simulink model "ofdm_txrx_alamouti"  (Simulink 6.3)
settings: Solver=ode45  StartTime=0.0  StopTime=45000  RelTol=1e-3
InitFcn:
  | ofdm_rx_alamouti_init
StartFcn:
  | ofdm_rx_alamouti_init
values from neighbouring files (symbols this model uses):
  CPLength = 16    [ofdm_tx_alamouti_init.m]
  CRCPolynomial = hex2dec('04c11db7')    [calcTxCRC.m]    (also in ofdm_tx_alamouti_init.m)
  hi = zeros(1,length(cci))    [ofdm_rx_alamouti_init.m]
  hr = zeros(1,length(ccr))    [ofdm_rx_alamouti_init.m]
  max_CP_length = 16    [ofdm_tx_alamouti_init.m]
  max_num_baseRateSymbols = 31    [ofdm_tx_alamouti_init.m]
  max_num_subcarriers = 64    [ofdm_tx_alamouti_init.m]
  max_num_trainingSymbols = 15    [ofdm_tx_alamouti_init.m]
  max_OFDM_symbols = 2047    [ofdm_tx_alamouti_init.m]
  modConstellation_qam16 = 0.75*[1 3 -1 -3]./3    [ofdm_tx_alamouti_init.m]
  modConstellation_qam256 = 0.9375*[3 1 5 7 11 9 13 15 -3 -1 -5 -7 -11 -9 -13 -15]./15    [ofdm_tx_alamouti_init.m]
  modConstellation_qam64 = 0.875*[3 1 5 7 -3 -1 -5 -7]./7    [ofdm_tx_alamouti_init.m]
  modConstellation_qpsk = [1 -1]./sqrt(2)    [ofdm_tx_alamouti_init.m]
  numBaseRateSymbols = 2    [ofdm_tx_alamouti_init.m]
  numFullRateSymbols = numFullRateSymbols + mod(numFullRateSymbols, 2)    [ofdm_tx_alamouti_init.m]
  numSubcarriers = 64    [ofdm_tx_alamouti_init.m]
  numTrainingSymbols = 4    [ofdm_tx_alamouti_init.m]
  pkt_crossCorr_thresh = 0.7    [ofdm_rx_alamouti_init.m]
  pkt_energy_thresh = 0    [ofdm_rx_alamouti_init.m]
  pkt_numPayloadBytes = 24+4+20+20+500    (= 568)    [ofdm_tx_alamouti_init.m]
  pktTiming_count_payloadStart = pktTiming_count_lsStart + pktTiming_ls_num*pktTiming_ls_length    [ofdm_rx_init_packetTimingControl.m]
  preamble = 6*[0 shortsyms_10 longsyms_2]    [ofdm_tx_alamouti_init.m]
  preamble_I = real(preamble)    [ofdm_tx_alamouti_init.m]
  preamble_Q = imag(preamble)    [ofdm_tx_alamouti_init.m]
  RAM_init_size = 4096    [ofdm_tx_alamouti_init.m]
  rx_longCorrCountLoad = 251-16    (= 235)    [ofdm_rx_alamouti_init.m]
  rx_longCorrThresh = 3000    [ofdm_rx_alamouti_init.m]
  RxDataScrambling_Seq = [40 198 78 63 82 173 102 245 48 111 172 115 147 230 216 93 72 65 62 2 205 242 122 90 128 83 105 97 73 10 5 252]    [ofdm_tx_alamouti_init.m]
  simOnly_numSamples = length(preamble)+( (numSubcarriers+CPLength)*(numTrainingSymbols + numBaseRateSymbols + numFullRateSymbols) )    [ofdm_tx_alamouti_init.m]
  train = [train train]    [ofdm_tx_alamouti_init.m]
  TxDataScrambling_Seq = [40 198 78 63 82 173 102 245 48 111 172 115 147 230 216 93 72 65 62 2 205 242 122 90 128 83 105 97 73 10 5 252]    [ofdm_tx_alamouti_init.m]
undefined symbols (not defined in the file or in its folder): CRC_table_gen, gcbh, interpFilter_4, xlBlockLoadCallback
libraries: xbsIndex_r4 x4373, dspmlti4 x4, dspsigops x4, commchan3 x2, simulink x2, commrflib2 x2, commsink2 x2, dspsrcs4 x1
(part 7 of 39)

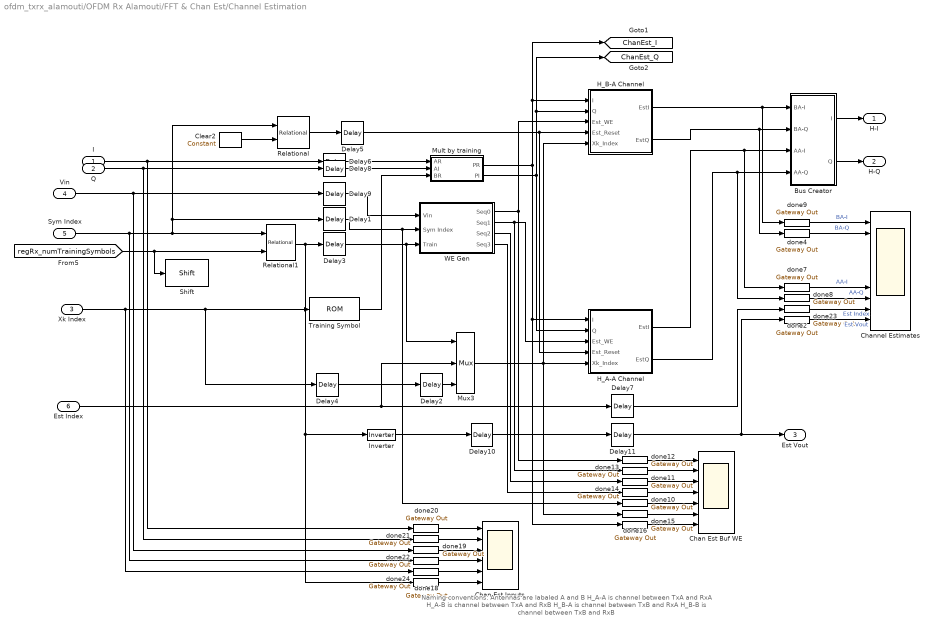
== system ofdm_txrx_alamouti/OFDM Rx Alamouti/FFT & Chan Est/Channel Estimation ==
  note: Naming conventions:
      | Antennas are labaled A and B
      | H_A-A is channel between TxA and RxA
      | H_A-B is channel between TxA and RxB
      | H_B-A is channel between TxB and RxA
      | H_B-B is channel between TxB and RxB
  I : Inport
  Q : Inport  Port=2
  Xk Index : Inport  Port=3
  Vin : Inport  Port=4
  Sym Index : Inport  Port=5
  Est Index : Inport  Port=6
  Bus Creator : Subsystem
  Chan Est Buf WE : Scope  NumInputPorts=7
      settings: TimeRange=2000  YMin=0~0~0~0~0~0~-0.4  YMax=1~1~1~1~30~70~0.4  SaveName=ScopeData21
  Chan Est Inputs : Scope  NumInputPorts=6
      settings: TimeRange=1800  YMin=-0.6~-0.4~0~5~0~-1  YMax=0.5~0.4~1~12~70~1  SaveName=ScopeData23
  Channel Estimates : Scope  NumInputPorts=10
      settings: TimeRange=3000  YMin=-1~-1~-1~-1~-1~-1~-1~-1~-1~0  YMax=1~1~1~1~1~1~1~1~1~1  SaveName=ScopeData24
  Clear2 : xbsIndex_r4/Constant  arith_type=Unsigned  const=1  n_bits=2  bin_pt=0  explicit_period=off  period=1  equ=P=C  opselect=C  inp2=PCIN>>17  opr=+  inp1=P  carry=CIN  dbl_ovrd=off  block_type=constant
      settings: block_version=VER_STRING_GOES_HERE
  Delay1 : xbsIndex_r4/Delay  en=off  latency=1  dbl_ovrd=off  reg_retiming=on  block_type=delay
      settings: infoedit=Hardware notes: A delay line is a chain, each link of which is an SRL16 followed by a flip-flop. If register retiming is enabled, the delay line is a chain of flip-flops.  xl_area=[0, 0, 0, 0, 0, 0, 0]  block_version=VER_STRING_GOES_HERE
  Delay{2,4..11} (9 alike) : xbsIndex_r4/Delay  en=off  latency=1  dbl_ovrd=off  reg_retiming=off  block_type=delay
      settings: infoedit=Hardware notes: A delay line is a chain, each link of which is an SRL16 followed by a flip-flop. If register retiming is enabled, the delay line is a chain of flip-flops.  xl_area=[0, 0, 0, 0, 0, 0, 0]  block_version=VER_STRING_GOES_HERE
  Delay3 : xbsIndex_r4/Delay  en=off  latency=1  dbl_ovrd=off  reg_retiming=on  block_type=delay
      settings: infoedit=Hardware notes: A delay line is a chain, each link of which is an SRL16 followed by a flip-flop. If register retiming is enabled, the delay line is a chain of flip-flops.  xl_area=[0, 0, 0, 0, 0, 0, 0]  block_version=VER_STRING_GOES_HERE
  H_A-A Channel : Subsystem
  H_B-A Channel : Subsystem
  Inverter : xbsIndex_r4/Inverter  en=off  latency=0  dbl_ovrd=off  block_type=inv
      settings: block_version=VER_STRING_GOES_HERE
  Mult by training : Subsystem
  Mux3 : xbsIndex_r4/Mux  inputs=2  en=off  latency=0  precision=Full  arith_type="Signed  (2's comp)"  n_bits=8  bin_pt=2  quantization=Truncate  overflow=Wrap  dbl_ovrd=off  block_type=mux
      settings: xl_area=[0, 0, 0, 0, 0, 0, 0]  block_version=VER_STRING_GOES_HERE
  Relational : xbsIndex_r4/Relational  mode=a<=b  en=off  latency=0  dbl_ovrd=off  block_type=relational
      settings: xl_area=[0, 0, 0, 0, 0, 0, 0]  block_version=VER_STRING_GOES_HERE
  Relational1 : xbsIndex_r4/Relational  mode=a<b  en=off  latency=0  dbl_ovrd=off  block_type=relational
      settings: xl_area=[3 0 0 6 0 0 0]  block_version=VER_STRING_GOES_HERE
  Shift : xbsIndex_r4/Shift  shift_dir=Left  shift_bits=1  en=off  latency=0  precision=User Defined  arith_type=Unsigned  n_bits=1+ceil(log2(max_num_trainingSymbols))  bin_pt=0  quantization=Truncate  overflow=Flag as error  dbl_ovrd=off  block_type=shift
      settings: block_version=VER_STRING_GOES_HERE
  Training Symbol : xbsIndex_r4/ROM  depth=length(train)/2  initVector=sign(train)  distributed_mem=Block RAM  rst=off  init_reg=0  en=off  latency=1  arith_type="Signed  (2's comp)"  n_bits=2  bin_pt=1  dbl_ovrd=off  optimize=Area  use_rpm=off  block_type=sprom
      settings: xl_area=[0, 0, 0, 0, 0, 0, 0]  block_version=VER_STRING_GOES_HERE
  WE Gen : Subsystem
  done{2,4,7..16,18..24} (19 alike) : xbsIndex_r4/Gateway Out  hdl_port=off  timing_constraint=None  locs_specified=off  LOCs={}  block_type=gatewayout
      settings: infoedit="Gateway out block.  Converts Xilinx fixed point inputs into ouputs of type Simulink integer, double, or fixed point.<P><P>Hardware notes:  In hardware these blocks become top level output ports or are discarded, depending on how they are configured."  xl_area=[0 0 0 0 1 0 0]  block_version=VER_STRING_GOES_HERE
  H-I : Outport
  H-Q : Outport  Port=2
  Est Vout : Outport  Port=3
  tag scope: ChanEst_I=global, ChanEst_Q=global
  wires:
  Training Symbol/1 -> Mult by training/3(BR)
  Delay4/1 -> Delay2/1
  I/1 -> Delay6/1, done20/1
  Q/1 -> Delay8/1, done21/1
  Sym Index/1 -> Relational1/1, Delay1/1, Relational/1, done22/1
  Xk Index/1 -> Delay4/1, Training Symbol/1, done18/1
  Clear2/1 -> Relational/2
  Relational/1 -> Delay5/1
  Mult by training/1(PR) -> done15/1, H_A-A Channel/1(I), H_B-A Channel/1(I), [ChanEst_I]
  Mux3/1 -> done16/1, H_A-A Channel/5(Xk_Index), H_B-A Channel/5(Xk_Index)
  Delay5/1 -> H_B-A Channel/4(Est_Reset), H_A-A Channel/4(Est_Reset)
  WE Gen/1(Seq0) -> H_B-A Channel/3(Est_WE), done12/1
  WE Gen/3(Seq2) -> done11/1
  WE Gen/4(Seq3) -> done14/1
  WE Gen/2(Seq1) -> done13/1, H_A-A Channel/3(Est_WE)
  Relational1/1 -> Delay3/1, done24/1, Inverter/1
  Mult by training/2(PI) -> H_B-A Channel/2(Q), [ChanEst_Q], H_A-A Channel/2(Q)
  done10/1 -> Chan Est Buf WE/5
  done12/1 -> Chan Est Buf WE/1
  done13/1 -> Chan Est Buf WE/2
  done11/1 -> Chan Est Buf WE/3
  done14/1 -> Chan Est Buf WE/4
  done16/1 -> Chan Est Buf WE/6
  done15/1 -> Chan Est Buf WE/7
  [regRx_numTrainingSymbols] -> Shift/1, Relational1/2
  Delay1/1 -> WE Gen/2(Sym Index), done10/1
  Delay3/1 -> WE Gen/3(Train), Mux3/1
  Delay6/1 -> Mult by training/1(AR)
  Delay8/1 -> Mult by training/2(AI)
  Vin/1 -> Delay9/1, done19/1
  Delay9/1 -> WE Gen/1(Vin)
  done18/1 -> Chan Est Inputs/5
  done20/1 -> Chan Est Inputs/1
  done21/1 -> Chan Est Inputs/2
  done19/1 -> Chan Est Inputs/3
  done22/1 -> Chan Est Inputs/4
  done24/1 -> Chan Est Inputs/6
  Delay7/1 -> done2/1
  Inverter/1 -> Delay10/1
  Delay11/1 -> done23/1, Est Vout/1
  H_B-A Channel/1(EstI) -> done9/1, Bus Creator/1(BA-I)
  H_B-A Channel/2(EstQ) -> done4/1, Bus Creator/2(BA-Q)
  H_A-A Channel/2(EstQ) -> done8/1, Bus Creator/4(AA-Q)
  ? -> Channel Estimates/6
  done4/1 -> Channel Estimates/2  "BA-Q"
  ? -> Channel Estimates/3
  ? -> Channel Estimates/4
  ? -> Channel Estimates/5
  done7/1 -> Channel Estimates/7  "AA-I"
  done8/1 -> Channel Estimates/8  "AA-Q"
  done2/1 -> Channel Estimates/9  "Est Index"
  done9/1 -> Channel Estimates/1  "BA-I"
  done23/1 -> Channel Estimates/10  "Est Vout"
  Bus Creator/1(I) -> H-I/1
  Bus Creator/2(Q) -> H-Q/1
  Delay10/1 -> Delay11/1
  Est Index/1 -> Delay7/1, Mux3/2
  Delay2/1 -> Mux3/3
  H_A-A Channel/1(EstI) -> done7/1, Bus Creator/3(AA-I)

== system ofdm_txrx_alamouti/OFDM Rx Alamouti/FFT & Chan Est/FFT ==
  xn_I : Inport
  xn_Q : Inport  Port=2
  Start : Inport  Port=3
  Constant3 : xbsIndex_r4/Constant  arith_type=Boolean  const=1  n_bits=1  bin_pt=0  explicit_period=off  period=1  equ=P=C  opselect=C  inp2=PCIN>>17  opr=+  inp1=P  carry=CIN  dbl_ovrd=off  block_type=constant
      settings: block_version=VER_STRING_GOES_HERE
  Constant4 : xbsIndex_r4/Constant  arith_type=Boolean  const=1  n_bits=1  bin_pt=0  explicit_period=off  period=1  equ=P=C  opselect=C  inp2=PCIN>>17  opr=+  inp1=P  carry=CIN  dbl_ovrd=off  block_type=constant
      settings: block_version=VER_STRING_GOES_HERE
  Constant6 : xbsIndex_r4/Constant  arith_type=Boolean  const=1  n_bits=1  bin_pt=0  explicit_period=off  period=1  equ=P=C  opselect=C  inp2=PCIN>>17  opr=+  inp1=P  carry=CIN  dbl_ovrd=off  block_type=constant
      settings: block_version=VER_STRING_GOES_HERE
  Convert : xbsIndex_r4/Convert  arith_type="Signed  (2's comp)"  n_bits=16  bin_pt=15  quantization=Truncate  overflow=Flag as error  latency=0  dbl_ovrd=off  pipeline=off  block_type=convert
      settings: block_version=VER_STRING_GOES_HERE
  Convert1 : xbsIndex_r4/Convert  arith_type="Signed  (2's comp)"  n_bits=16  bin_pt=15  quantization=Truncate  overflow=Flag as error  latency=0  dbl_ovrd=off  pipeline=off  block_type=convert
      settings: block_version=VER_STRING_GOES_HERE
  FFT Input : Scope  NumInputPorts=4
      settings: TimeRange=4000  YMin=-0.1~-0.2~0~0  YMax=0.15~0.8~1~80  SaveName=ScopeData14
  FFT Output : Scope  NumInputPorts=6
      settings: TimeRange=12000  YMin=-0.75~-0.75~0~0~0~-1  YMax=0.75~0.75~70~1~1~1  SaveName=ScopeData15
  FFTx : xbsIndex_r4/FFT v3_1   arch=Pipelined Streaming I/O  npts=64  scaling=Scaled  rounding=Convergent Rounding  tf_width=16  en_n=off  has_ovflo=on  rst=on  en=off  op_order=Natural Order  dbl_ovrd=off  explicit_period=off  period=1  use_dsp48=off  twiddle_mem_type=Block RAM  data_mem_type=Block RAM  num_bram_stages=3  block_type=fftx
      settings: infoedit=Devices Supported: Virtex-4, Virtex-II, Virtex-IIPro, Spartan-3 and Spartan-3E  xl_area=[0, 0, 0, 0, 0, 0, 0]  block_version=VER_STRING_GOES_HERE
  done{1..10} (10 alike) : xbsIndex_r4/Gateway Out  hdl_port=off  timing_constraint=None  locs_specified=off  LOCs={}  block_type=gatewayout
      settings: infoedit="Gateway out block.  Converts Xilinx fixed point inputs into ouputs of type Simulink integer, double, or fixed point.<P><P>Hardware notes:  In hardware these blocks become top level output ports or are discarded, depending on how they are configured."  xl_area=[0 0 0 0 1 0 0]  block_version=VER_STRING_GOES_HERE
  xk_I : Outport
  xk_Q : Outport  Port=2
  xk_Index : Outport  Port=3
  Vout : Outport  Port=4
  Done : Outport  Port=5
  xn_Index : Outport  Port=6
  wires:
  Constant3/1 -> FFTx/4
  Constant4/1 -> FFTx/5
  [rx_global_reset] -> FFTx/8
  xn_I/1 -> Convert/1
  FFTx/1 -> xk_I/1, done8/1
  FFTx/2 -> xk_Q/1, done9/1
  xn_Q/1 -> Convert1/1
  FFTx/3 -> xn_Index/1, done6/1
  Start/1 -> FFTx/3, done3/1
  FFTx/4 -> xk_Index/1, done7/1
  FFTx/7 -> Vout/1, done10/1
  Convert/1 -> FFTx/1, done4/1
  Convert1/1 -> FFTx/2, done5/1
  done4/1 -> FFT Input/1
  done5/1 -> FFT Input/2
  done3/1 -> FFT Input/3
  done6/1 -> FFT Input/4
  done8/1 -> FFT Output/1
  done9/1 -> FFT Output/2
  done7/1 -> FFT Output/3
  done10/1 -> FFT Output/4
  done2/1 -> FFT Output/5
  FFTx/9 -> done2/1, Done/1
  done1/1 -> FFT Output/6
  Constant6/1 -> FFTx/7
  [Rx_FFTScaling] -> FFTx/6
  FFTx/10 -> done1/1

== system ofdm_txrx_alamouti/OFDM Rx Alamouti/FFT & Chan Est/Input Buffers ==
  AntA I : Inport
  AntA Q : Inport  Port=2
  We : Inport  Port=3
  Wr Addr : Inport  Port=4
  Rd Addr : Inport  Port=5
  Ant Sel : Inport  Port=6
  Wr Slot : Inport  Port=7
  Antenna A RAM : Subsystem
  Antenna B RAM : Subsystem
  Inverter1 : xbsIndex_r4/Inverter  en=off  latency=0  dbl_ovrd=off  block_type=inv
      settings: block_version=VER_STRING_GOES_HERE
  Logical : xbsIndex_r4/Logical  logical_function=AND  inputs=2  en=off  latency=0  precision=Full  arith_type=Unsigned  n_bits=16  bin_pt=0  align_bp=on  dbl_ovrd=off  block_type=logical
      settings: block_version=8.2.02
  Logical1 : xbsIndex_r4/Logical  logical_function=AND  inputs=2  en=off  latency=0  precision=Full  arith_type=Unsigned  n_bits=16  bin_pt=0  align_bp=on  dbl_ovrd=off  block_type=logical
      settings: block_version=8.2.02
  Mux : xbsIndex_r4/Mux  inputs=2  en=off  latency=1  precision=Full  arith_type=Unsigned  n_bits=16  bin_pt=14  quantization=Truncate  overflow=Wrap  dbl_ovrd=off  block_type=mux
      settings: block_version=VER_STRING_GOES_HERE
  Mux1 : xbsIndex_r4/Mux  inputs=2  en=off  latency=1  precision=Full  arith_type=Unsigned  n_bits=16  bin_pt=14  quantization=Truncate  overflow=Wrap  dbl_ovrd=off  block_type=mux
      settings: block_version=VER_STRING_GOES_HERE
  Register : xbsIndex_r4/Register  init=0  rst=off  en=off  dbl_ovrd=off  block_type=register
      settings: block_version=VER_STRING_GOES_HERE
  Register1 : xbsIndex_r4/Register  init=0  rst=off  en=off  dbl_ovrd=off  block_type=register
      settings: block_version=VER_STRING_GOES_HERE
  Rx Buffers : Scope  NumInputPorts=6
      settings: TimeRange=2500  YMin=-0.015~-0.015~0~0~0~-5  YMax=0.015~0.015~80~80~1~5  SaveName=ScopeData19
  Rx Buffers Out : Scope  NumInputPorts=7
      settings: TimeRange=2500  YMin=-0.2~-0.7~0~-0.2~-0.7~-0.2~0  YMax=0.5~0.3~0.5~0.5~0~0.25~1  SaveName=ScopeData32
  done{1..13} (13 alike) : xbsIndex_r4/Gateway Out  hdl_port=off  timing_constraint=None  locs_specified=off  LOCs={}  block_type=gatewayout
      settings: infoedit="Gateway out block.  Converts Xilinx fixed point inputs into ouputs of type Simulink integer, double, or fixed point.<P><P>Hardware notes:  In hardware these blocks become top level output ports or are discarded, depending on how they are configured."  xl_area=[0 0 0 0 1 0 0]  block_version=VER_STRING_GOES_HERE
  " I" : Outport
  " Q" : Outport  Port=2
  wires:
  AntA I/1 -> Antenna A RAM/1(I), done8/1, Antenna B RAM/1(I)
  AntA Q/1 -> Antenna A RAM/2(Q), done9/1, Antenna B RAM/2(Q)
  Wr Addr/1 -> Antenna A RAM/4(Wr Addr), done7/1, Antenna B RAM/4(Wr Addr)
  Rd Addr/1 -> Antenna A RAM/5(Rd Addr), done10/1, Antenna B RAM/5(Rd Addr)
  Antenna A RAM/1(" I") -> Mux/2, done12/1
  Antenna A RAM/2(" Q") -> Mux1/2, done6/1
  Antenna B RAM/2(" Q") -> Mux1/3, done5/1
  Antenna B RAM/1(" I") -> Mux/3, done4/1
  Ant Sel/1 -> Mux/1, Mux1/1, done11/1
  Mux/1 -> Register/1
  Mux1/1 -> Register1/1
  Register/1 -> " I"/1, done3/1
  Register1/1 -> " Q"/1, done13/1
  done8/1 -> Rx Buffers/1  "AntA I"
  done9/1 -> Rx Buffers/2  "AntQ Q"
  done7/1 -> Rx Buffers/3  "Write Addr"
  done10/1 -> Rx Buffers/4  "Rd Addr"
  done2/1 -> Rx Buffers/5  "Write En A"
  done1/1 -> Rx Buffers/6  "Write En B"
  done11/1 -> Rx Buffers Out/1
  done12/1 -> Rx Buffers Out/2
  done6/1 -> Rx Buffers Out/3
  done4/1 -> Rx Buffers Out/4
  done5/1 -> Rx Buffers Out/5
  done3/1 -> Rx Buffers Out/6
  done13/1 -> Rx Buffers Out/7
  We/1 -> Logical/1, Logical1/1
  Wr Slot/1 -> Logical/2, Inverter1/1
  Inverter1/1 -> Logical1/2
  Logical/1 -> Antenna A RAM/3(We), done2/1
  Logical1/1 -> Antenna B RAM/3(We), done1/1

== system ofdm_txrx_alamouti/OFDM Rx Alamouti/FFT & Chan Est/Output Buffers ==
  I : Inport
  Q : Inport  Port=2
  Wr Addr : Inport  Port=3
  FFT Vout : Inport  Port=4
  SymIndex : Inport  Port=5
  Rd Addr : Inport  Port=6
  AntA Buffer : Subsystem
  AntB Buffer : Subsystem
  Bus Creator1 : BusCreator  Inputs=2
  Bus Creator2 : BusCreator  Inputs=2
  FFT Output Buffers : Scope  NumInputPorts=10
      settings: TimeRange=5000  YMin=-0.5~-0.5~0~0~0~0~-0.5~-1~0~0  YMax=0.5~0.5~100~1~1~100~0.5~1~0.02~1  SaveName=ScopeData53
  Inverter : xbsIndex_r4/Inverter  en=off  latency=0  dbl_ovrd=off  block_type=inv
      settings: block_version=VER_STRING_GOES_HERE
  LSB : xbsIndex_r4/Slice  nbits=1  boolean_output=on  mode=Upper Bit Location + Width  bit1=0  base1=LSB of Input  bit0=0  base0=LSB of Input  dbl_ovrd=off  block_type=slice
      settings: infoedit="Extracts a given range of bits from each input sample and presents it at the output.  The output type is ordinarily unsigned with binary point at zero, but can be Boolean when the slice is one bit wide.<P><P>Hardware notes: In hardware this block costs nothing."  block_version=VER_STRING_GOES_HERE
  Logical1 : xbsIndex_r4/Logical  logical_function=AND  inputs=2  en=off  latency=0  precision=Full  arith_type=Unsigned  n_bits=8  bin_pt=2  align_bp=on  dbl_ovrd=off  block_type=logical
      settings: xl_area=[0, 0, 0, 0, 0, 0, 0]  block_version=VER_STRING_GOES_HERE
  Logical2 : xbsIndex_r4/Logical  logical_function=AND  inputs=2  en=off  latency=0  precision=Full  arith_type=Unsigned  n_bits=8  bin_pt=2  align_bp=on  dbl_ovrd=off  block_type=logical
      settings: xl_area=[0, 0, 0, 0, 0, 0, 0]  block_version=VER_STRING_GOES_HERE
  done{1..10} (10 alike) : xbsIndex_r4/Gateway Out  hdl_port=off  timing_constraint=None  locs_specified=off  LOCs={}  block_type=gatewayout
      settings: infoedit="Gateway out block.  Converts Xilinx fixed point inputs into ouputs of type Simulink integer, double, or fixed point.<P><P>Hardware notes:  In hardware these blocks become top level output ports or are discarded, depending on how they are configured."  xl_area=[0 0 0 0 1 0 0]  block_version=VER_STRING_GOES_HERE
  " I" : Outport
  " Q" : Outport  Port=2
  wires:
  Rd Addr/1 -> AntA Buffer/5(Rd Addr), AntB Buffer/5(Rd Addr), done1/1
  SymIndex/1 -> LSB/1
  Logical2/1 -> AntA Buffer/3(We), done2/1
  Logical1/1 -> AntB Buffer/3(We), done10/1
  LSB/1 -> Inverter/1, Logical2/1
  AntB Buffer/1(" I") -> done3/1, Bus Creator1/2
  AntB Buffer/2(" Q") -> done4/1, Bus Creator2/2
  AntA Buffer/1(" I") -> done5/1, Bus Creator1/1
  AntA Buffer/2(" Q") -> done6/1, Bus Creator2/1
  FFT Vout/1 -> Logical2/2, Logical1/2
  Wr Addr/1 -> AntA Buffer/4(Wr Addr), AntB Buffer/4(Wr Addr), done7/1
  I/1 -> AntB Buffer/1(I), AntA Buffer/1(I), done8/1
  Q/1 -> AntB Buffer/2(Q), AntA Buffer/2(Q), done9/1
  done8/1 -> FFT Output Buffers/1  "I"
  done9/1 -> FFT Output Buffers/2  "Q"
  done7/1 -> FFT Output Buffers/3  "Wr Addr"
  done10/1 -> FFT Output Buffers/5  "B WE"
  done2/1 -> FFT Output Buffers/4  "A WE"
  done1/1 -> FFT Output Buffers/6  "Rd Addr"
  done3/1 -> FFT Output Buffers/9  "B_I"
  done4/1 -> FFT Output Buffers/10  "B_Q"
  done5/1 -> FFT Output Buffers/7  "A_I"
  done6/1 -> FFT Output Buffers/8  "A_Q"
  Bus Creator1/1 -> " I"/1
  Bus Creator2/1 -> " Q"/1
  Inverter/1 -> Logical1/1

== system ofdm_txrx_alamouti/OFDM Rx Alamouti/FFT & Chan Est/Symbol Counter ==
  note: SR Latch enables counter after the first symbol. This keeps
      | the symbol index at zero after the first FFT finishes so that
      | the symbol index, like xn and xk_index, is zero-indexed.
  FFT Done : Inport
  Payload : Inport  Port=2
  Counter : xbsIndex_r4/Counter  cnt_type=Free Running  cnt_to=Inf  operation=Up  start_count=0  cnt_by_val=1  arith_type=Unsigned  n_bits=ceil(log2(max_OFDM_symbols+1))  bin_pt=0  load_pin=off  rst=on  en=on  explicit_period=off  period=1  dbl_ovrd=off  use_behavioral_HDL=off  use_rpm=on  block_type=counter
      settings: xl_area=[6 12 0 12 0 0 0]  block_version=VER_STRING_GOES_HERE
  Logical1 : xbsIndex_r4/Logical  logical_function=AND  inputs=2  en=off  latency=0  precision=Full  arith_type=Unsigned  n_bits=8  bin_pt=2  align_bp=on  dbl_ovrd=off  block_type=logical
      settings: xl_area=[0, 0, 0, 0, 0, 0, 0]  block_version=VER_STRING_GOES_HERE
  Logical2 : xbsIndex_r4/Logical  logical_function=OR  inputs=2  en=off  latency=0  precision=Full  arith_type=Unsigned  n_bits=8  bin_pt=2  align_bp=on  dbl_ovrd=off  block_type=logical
      settings: xl_area=[4 0 0 4 0 0 0]  block_version=VER_STRING_GOES_HERE
  Posedge Det : Subsystem
  S-R Latch : Subsystem
  Sym Index : Outport
  wires:
  FFT Done/1 -> Logical1/1, S-R Latch/1(S)
  Counter/1 -> Sym Index/1
  Logical1/1 -> Counter/2
  S-R Latch/1(D) -> Logical1/2
  Logical2/1 -> Counter/1, S-R Latch/2(R)
  Posedge Det/1(Q) -> Logical2/1
  [rx_global_reset] -> Logical2/2
  Payload/1 -> Posedge Det/1(D)

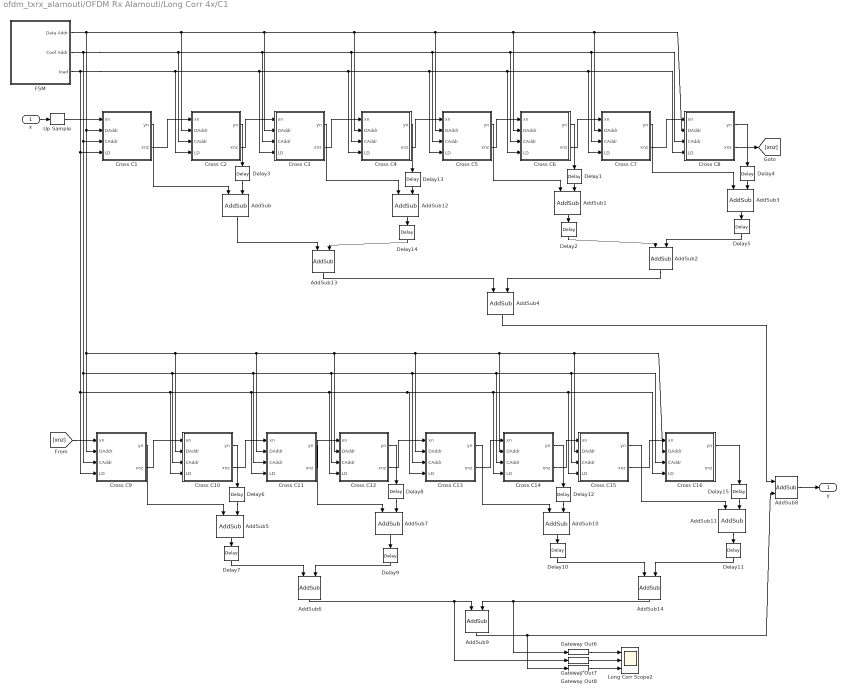
== system ofdm_txrx_alamouti/OFDM Rx Alamouti/Long Corr 4x/C1 ==
  x : Inport
  AddSub : xbsIndex_r4/AddSub  Orientation=down  mode=Addition  use_carryin=off  use_carryout=off  en=off  latency=0  precision=Full  arith_type=Unsigned  n_bits=8  bin_pt=2  quantization=Truncate  overflow=Wrap  dbl_ovrd=off  use_behavioral_HDL=on  pipelined=off  use_rpm=off  block_type=addsub
      settings: xl_area=[3 0 0 4 0 0 0]  block_version=VER_STRING_GOES_HERE
  AddSub{1..7,9..14} (13 alike) : xbsIndex_r4/AddSub  Orientation=down  mode=Addition  use_carryin=off  use_carryout=off  en=off  latency=1  precision=Full  arith_type=Unsigned  n_bits=8  bin_pt=2  quantization=Truncate  overflow=Wrap  dbl_ovrd=off  use_behavioral_HDL=on  pipelined=off  use_rpm=off  block_type=addsub
      AddSub1 settings: xl_area=[3 5 0 4 0 0 0]  block_version=VER_STRING_GOES_HERE
      AddSub10 settings: xl_area=[3 5 0 4 0 0 0]  block_version=VER_STRING_GOES_HERE
      AddSub11 settings: xl_area=[3 5 0 4 0 0 0]  block_version=VER_STRING_GOES_HERE
      AddSub12 settings: xl_area=[3 5 0 4 0 0 0]  block_version=VER_STRING_GOES_HERE
      AddSub13 settings: xl_area=[3 6 0 5 0 0 0]  block_version=VER_STRING_GOES_HERE
      AddSub14 settings: xl_area=[3 6 0 5 0 0 0]  block_version=VER_STRING_GOES_HERE
      AddSub2 settings: xl_area=[3 6 0 5 0 0 0]  block_version=VER_STRING_GOES_HERE
      AddSub3 settings: xl_area=[3 5 0 4 0 0 0]  block_version=VER_STRING_GOES_HERE
      AddSub4 settings: xl_area=[4 7 0 6 0 0 0]  block_version=VER_STRING_GOES_HERE
      AddSub5 settings: xl_area=[3 5 0 4 0 0 0]  block_version=VER_STRING_GOES_HERE
      AddSub6 settings: xl_area=[3 6 0 5 0 0 0]  block_version=VER_STRING_GOES_HERE
      AddSub7 settings: xl_area=[3 5 0 4 0 0 0]  block_version=VER_STRING_GOES_HERE
      AddSub9 settings: xl_area=[4 7 0 6 0 0 0]  block_version=VER_STRING_GOES_HERE
  AddSub8 : xbsIndex_r4/AddSub  mode=Addition  use_carryin=off  use_carryout=off  en=off  latency=1  precision=Full  arith_type=Unsigned  n_bits=8  bin_pt=2  quantization=Truncate  overflow=Wrap  dbl_ovrd=off  use_behavioral_HDL=on  pipelined=off  use_rpm=off  block_type=addsub
      settings: xl_area=[4 8 0 7 0 0 0]  block_version=VER_STRING_GOES_HERE
  Cross C1 : Subsystem  mask{coef2=h(1:N)}
      mask prompts: coef2 "Coefficients"
  Cross C10 : Subsystem  mask{coef2=h(9*N+1:10*N)}
      mask prompts: coef2 "Coefficients"
  Cross C11 : Subsystem  mask{coef2=h(10*N+1:11*N)}
      mask prompts: coef2 "Coefficients"
  Cross C12 : Subsystem  mask{coef2=h(11*N+1:12*N)}
      mask prompts: coef2 "Coefficients"
  Cross C13 : Subsystem  mask{coef2=h(12*N+1:13*N)}
      mask prompts: coef2 "Coefficients"
  Cross C14 : Subsystem  mask{coef2=h(13*N+1:14*N)}
      mask prompts: coef2 "Coefficients"
  Cross C15 : Subsystem  mask{coef2=h(14*N+1:15*N)}
      mask prompts: coef2 "Coefficients"
  Cross C16 : Subsystem  mask{coef2=h(15*N+1:16*N)}
      mask prompts: coef2 "Coefficients"
  Cross C2 : Subsystem  mask{coef2=h(1*N+1:2*N)}
      mask prompts: coef2 "Coefficients"
  Cross C3 : Subsystem  mask{coef2=h(2*N+1:3*N)}
      mask prompts: coef2 "Coefficients"
  Cross C4 : Subsystem  mask{coef2=h(3*N+1:4*N)}
      mask prompts: coef2 "Coefficients"
  Cross C5 : Subsystem  mask{coef2=h(4*N+1:5*N)}
      mask prompts: coef2 "Coefficients"
  Cross C6 : Subsystem  mask{coef2=h(5*N+1:6*N)}
      mask prompts: coef2 "Coefficients"
  Cross C7 : Subsystem  mask{coef2=h(6*N+1:7*N)}
      mask prompts: coef2 "Coefficients"
  Cross C8 : Subsystem  mask{coef2=h(7*N+1:8*N)}
      mask prompts: coef2 "Coefficients"
  Cross C9 : Subsystem  mask{coef2=h(8*N+1:9*N)}
      mask prompts: coef2 "Coefficients"
  Delay{1,3..4,6,8,12..13,15} (8 alike) : xbsIndex_r4/Delay  Orientation=down  en=off  latency=N-1  dbl_ovrd=off  reg_retiming=off  block_type=delay
      settings: infoedit=Hardware notes: A delay line is a chain, each link of which is an SRL16 followed by a flip-flop. If register retiming is enabled, the delay line is a chain of flip-flops.  xl_area=[2 4 0 4 0 0 0]  block_version=VER_STRING_GOES_HERE
  Delay10 : xbsIndex_r4/Delay  Orientation=down  en=off  latency=((N-1)*12)-1  dbl_ovrd=off  reg_retiming=off  block_type=delay
      settings: infoedit=Hardware notes: A delay line is a chain, each link of which is an SRL16 followed by a flip-flop. If register retiming is enabled, the delay line is a chain of flip-flops.  xl_area=[9 15 0 15 0 0 0]  block_version=VER_STRING_GOES_HERE
  Delay11 : xbsIndex_r4/Delay  Orientation=down  en=off  latency=((N-1)*14)-1  dbl_ovrd=off  reg_retiming=off  block_type=delay
      settings: infoedit=Hardware notes: A delay line is a chain, each link of which is an SRL16 followed by a flip-flop. If register retiming is enabled, the delay line is a chain of flip-flops.  xl_area=[9 15 0 15 0 0 0]  block_version=VER_STRING_GOES_HERE
  Delay14 : xbsIndex_r4/Delay  Orientation=down  en=off  latency=((N-1)*2)-1  dbl_ovrd=off  reg_retiming=off  block_type=delay
      settings: infoedit=Hardware notes: A delay line is a chain, each link of which is an SRL16 followed by a flip-flop. If register retiming is enabled, the delay line is a chain of flip-flops.  xl_area=[3 5 0 5 0 0 0]  block_version=VER_STRING_GOES_HERE
  Delay2 : xbsIndex_r4/Delay  Orientation=down  en=off  latency=((N-1)*4)-1  dbl_ovrd=off  reg_retiming=off  block_type=delay
      settings: infoedit=Hardware notes: A delay line is a chain, each link of which is an SRL16 followed by a flip-flop. If register retiming is enabled, the delay line is a chain of flip-flops.  xl_area=[3 5 0 5 0 0 0]  block_version=VER_STRING_GOES_HERE
  Delay5 : xbsIndex_r4/Delay  Orientation=down  en=off  latency=((N-1)*6)-1  dbl_ovrd=off  reg_retiming=off  block_type=delay
      settings: infoedit=Hardware notes: A delay line is a chain, each link of which is an SRL16 followed by a flip-flop. If register retiming is enabled, the delay line is a chain of flip-flops.  xl_area=[6 10 0 10 0 0 0]  block_version=VER_STRING_GOES_HERE
  Delay7 : xbsIndex_r4/Delay  Orientation=down  en=off  latency=((N-1)*8)-1  dbl_ovrd=off  reg_retiming=off  block_type=delay
      settings: infoedit=Hardware notes: A delay line is a chain, each link of which is an SRL16 followed by a flip-flop. If register retiming is enabled, the delay line is a chain of flip-flops.  xl_area=[6 10 0 10 0 0 0]  block_version=VER_STRING_GOES_HERE
  Delay9 : xbsIndex_r4/Delay  Orientation=down  en=off  latency=((N-1)*10)-1  dbl_ovrd=off  reg_retiming=off  block_type=delay
      settings: infoedit=Hardware notes: A delay line is a chain, each link of which is an SRL16 followed by a flip-flop. If register retiming is enabled, the delay line is a chain of flip-flops.  xl_area=[9 15 0 15 0 0 0]  block_version=VER_STRING_GOES_HERE
  FSM : Subsystem
  Gateway Out6 : xbsIndex_r4/Gateway Out  hdl_port=off  timing_constraint=None  locs_specified=off  LOCs={}  block_type=gatewayout
      settings: infoedit="Gateway out block.  Converts Xilinx fixed point inputs into ouputs of type Simulink integer, double, or fixed point.<P><P>Hardware notes:  In hardware these blocks become top level output ports or are discarded, depending on how they are configured."  xl_area=[0 0 0 0 0 0 0]  block_version=VER_STRING_GOES_HERE
  Gateway Out7 : xbsIndex_r4/Gateway Out  hdl_port=off  timing_constraint=None  locs_specified=off  LOCs={}  block_type=gatewayout
      settings: infoedit="Gateway out block.  Converts Xilinx fixed point inputs into ouputs of type Simulink integer, double, or fixed point.<P><P>Hardware notes:  In hardware these blocks become top level output ports or are discarded, depending on how they are configured."  xl_area=[0 0 0 0 0 0 0]  block_version=VER_STRING_GOES_HERE
  Gateway Out8 : xbsIndex_r4/Gateway Out  hdl_port=off  timing_constraint=None  locs_specified=off  LOCs={}  block_type=gatewayout
      settings: infoedit="Gateway out block.  Converts Xilinx fixed point inputs into ouputs of type Simulink integer, double, or fixed point.<P><P>Hardware notes:  In hardware these blocks become top level output ports or are discarded, depending on how they are configured."  xl_area=[0 0 0 0 0 0 0]  block_version=VER_STRING_GOES_HERE
  Long Corr Scope2 : Scope  NumInputPorts=3
      settings: YMin=-0.35~-0.175~0  YMax=0.35~0.225~1300  SaveName=ScopeData8
  Up Sample : xbsIndex_r4/Up Sample  sample_ratio=4  copy_samples=on  dbl_ovrd=off  block_type=usamp
      settings: infoedit="Up samples input data.  Inserted values  can be zeros or copies of the most recent input sample.<P><P>Hardware notes: No hardware is needed if inserted values are copies of the input sample; otherwise, a mux and single bit flip-flop are used."  block_version=8.2.02
  y : Outport
  wires:
  AddSub9/1 -> Gateway Out8/1, AddSub8/2
  AddSub6/1 -> AddSub9/1, Gateway Out7/1
  Cross C13/1(yn) -> AddSub10/1
  AddSub8/1 -> y/1
  Delay9/1 -> AddSub6/2
  Delay7/1 -> AddSub6/1
  Cross C12/1(yn) -> Delay8/1
  AddSub7/1 -> Delay9/1
  Delay8/1 -> AddSub7/2
  Cross C11/1(yn) -> AddSub7/1
  AddSub4/1 -> AddSub8/1
  AddSub2/1 -> AddSub4/2
  AddSub13/1 -> AddSub4/1
  Delay5/1 -> AddSub2/2
  Delay2/1 -> AddSub2/1
  AddSub/1 -> AddSub13/1
  Delay14/1 -> AddSub13/2
  Cross C8/2(xnz) -> [xnz]
  Cross C10/1(yn) -> Delay6/1
  AddSub5/1 -> Delay7/1
  Delay6/1 -> AddSub5/2
  Cross C9/1(yn) -> AddSub5/1
  Cross C8/1(yn) -> Delay4/1
  Cross C7/1(yn) -> AddSub3/1
  AddSub3/1 -> Delay5/1
  Delay4/1 -> AddSub3/2
  Cross C7/2(xnz) -> Cross C8/1(xn)
  Cross C6/1(yn) -> Delay1/1
  AddSub1/1 -> Delay2/1
  Delay1/1 -> AddSub1/2
  Cross C5/1(yn) -> AddSub1/1
  Cross C1/1(yn) -> AddSub/1
  Delay3/1 -> AddSub/2
  AddSub12/1 -> Delay14/1
  Delay13/1 -> AddSub12/2
  Cross C12/2(xnz) -> Cross C13/1(xn)
  Cross C11/2(xnz) -> Cross C12/1(xn)
  Cross C10/2(xnz) -> Cross C11/1(xn)
  Cross C9/2(xnz) -> Cross C10/1(xn)
  [xnz] -> Cross C9/1(xn)
  Cross C6/2(xnz) -> Cross C7/1(xn)
  Cross C5/2(xnz) -> Cross C6/1(xn)
  Cross C4/1(yn) -> Delay13/1
  Cross C4/2(xnz) -> Cross C5/1(xn)
  Cross C3/1(yn) -> AddSub12/1
  Cross C3/2(xnz) -> Cross C4/1(xn)
  Cross C2/2(xnz) -> Cross C3/1(xn)
  FSM/3(load) -> Cross C15/4(LD), Cross C16/4(LD), Cross C14/4(LD), Cross C13/4(LD), Cross C12/4(LD), Cross C11/4(LD), Cross C10/4(LD), Cross C9/4(LD), Cross C1/4(LD), Cross C7/4(LD), Cross C8/4(LD), Cross C6/4(LD), Cross C5/4(LD), Cross C4/4(LD), Cross C3/4(LD), Cross C2/4(LD)
  FSM/2(Coef Addr) -> Cross C15/3(CAddr), Cross C16/3(CAddr), Cross C14/3(CAddr), Cross C13/3(CAddr), Cross C12/3(CAddr), Cross C11/3(CAddr), Cross C10/3(CAddr), Cross C9/3(CAddr), Cross C1/3(CAddr), Cross C7/3(CAddr), Cross C8/3(CAddr), Cross C6/3(CAddr), Cross C5/3(CAddr), Cross C4/3(CAddr), Cross C3/3(CAddr), Cross C2/3(CAddr)
  FSM/1(Data Addr) -> Cross C15/2(DAddr), Cross C16/2(DAddr), Cross C14/2(DAddr), Cross C13/2(DAddr), Cross C12/2(DAddr), Cross C11/2(DAddr), Cross C10/2(DAddr), Cross C9/2(DAddr), Cross C1/2(DAddr), Cross C7/2(DAddr), Cross C8/2(DAddr), Cross C6/2(DAddr), Cross C5/2(DAddr), Cross C4/2(DAddr), Cross C3/2(DAddr), Cross C2/2(DAddr)
  Cross C1/2(xnz) -> Cross C2/1(xn)
  Cross C2/1(yn) -> Delay3/1
  x/1 -> Up Sample/1
  Cross C13/2(xnz) -> Cross C14/1(xn)
  Cross C14/2(xnz) -> Cross C15/1(xn)
  Cross C15/2(xnz) -> Cross C16/1(xn)
  Cross C15/1(yn) -> AddSub11/1
  Cross C14/1(yn) -> Delay12/1
  Cross C16/1(yn) -> Delay15/1
  Delay12/1 -> AddSub10/2
  Delay15/1 -> AddSub11/2
  AddSub10/1 -> Delay10/1
  AddSub11/1 -> Delay11/1
  Delay10/1 -> AddSub14/1
  Delay11/1 -> AddSub14/2
  AddSub14/1 -> AddSub9/2, Gateway Out6/1
  Gateway Out6/1 -> Long Corr Scope2/1
  Gateway Out7/1 -> Long Corr Scope2/2
  Gateway Out8/1 -> Long Corr Scope2/3
  Up Sample/1 -> Cross C1/1(xn)

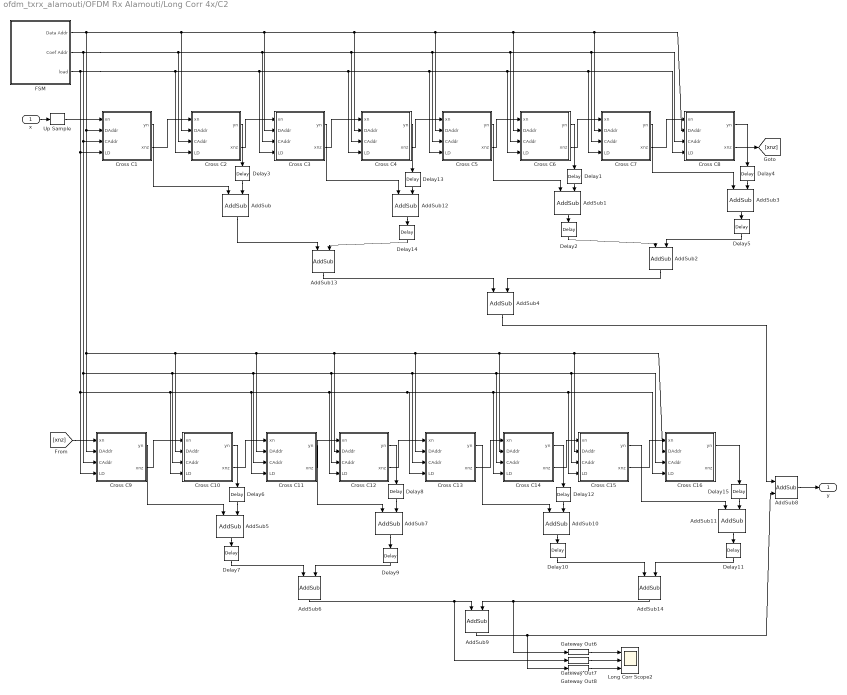
== system ofdm_txrx_alamouti/OFDM Rx Alamouti/Long Corr 4x/C2 ==
  x : Inport
  AddSub : xbsIndex_r4/AddSub  Orientation=down  mode=Addition  use_carryin=off  use_carryout=off  en=off  latency=0  precision=Full  arith_type=Unsigned  n_bits=8  bin_pt=2  quantization=Truncate  overflow=Wrap  dbl_ovrd=off  use_behavioral_HDL=on  pipelined=off  use_rpm=off  block_type=addsub
      settings: xl_area=[3 0 0 4 0 0 0]  block_version=VER_STRING_GOES_HERE
  AddSub{1..7,9..14} (13 alike) : xbsIndex_r4/AddSub  Orientation=down  mode=Addition  use_carryin=off  use_carryout=off  en=off  latency=1  precision=Full  arith_type=Unsigned  n_bits=8  bin_pt=2  quantization=Truncate  overflow=Wrap  dbl_ovrd=off  use_behavioral_HDL=on  pipelined=off  use_rpm=off  block_type=addsub
      AddSub1 settings: xl_area=[3 5 0 4 0 0 0]  block_version=VER_STRING_GOES_HERE
      AddSub10 settings: xl_area=[3 5 0 4 0 0 0]  block_version=VER_STRING_GOES_HERE
      AddSub11 settings: xl_area=[3 5 0 4 0 0 0]  block_version=VER_STRING_GOES_HERE
      AddSub12 settings: xl_area=[3 5 0 4 0 0 0]  block_version=VER_STRING_GOES_HERE
      AddSub13 settings: xl_area=[3 6 0 5 0 0 0]  block_version=VER_STRING_GOES_HERE
      AddSub14 settings: xl_area=[3 6 0 5 0 0 0]  block_version=VER_STRING_GOES_HERE
      AddSub2 settings: xl_area=[3 6 0 5 0 0 0]  block_version=VER_STRING_GOES_HERE
      AddSub3 settings: xl_area=[3 5 0 4 0 0 0]  block_version=VER_STRING_GOES_HERE
      AddSub4 settings: xl_area=[4 7 0 6 0 0 0]  block_version=VER_STRING_GOES_HERE
      AddSub5 settings: xl_area=[3 5 0 4 0 0 0]  block_version=VER_STRING_GOES_HERE
      AddSub6 settings: xl_area=[3 6 0 5 0 0 0]  block_version=VER_STRING_GOES_HERE
      AddSub7 settings: xl_area=[3 5 0 4 0 0 0]  block_version=VER_STRING_GOES_HERE
      AddSub9 settings: xl_area=[4 7 0 6 0 0 0]  block_version=VER_STRING_GOES_HERE
  AddSub8 : xbsIndex_r4/AddSub  mode=Addition  use_carryin=off  use_carryout=off  en=off  latency=1  precision=Full  arith_type=Unsigned  n_bits=8  bin_pt=2  quantization=Truncate  overflow=Wrap  dbl_ovrd=off  use_behavioral_HDL=on  pipelined=off  use_rpm=off  block_type=addsub
      settings: xl_area=[4 8 0 7 0 0 0]  block_version=VER_STRING_GOES_HERE
  Cross C1 : Subsystem  mask{coef2=h(1:N)}
      mask prompts: coef2 "Coefficients"
  Cross C10 : Subsystem  mask{coef2=h(9*N+1:10*N)}
      mask prompts: coef2 "Coefficients"
  Cross C11 : Subsystem  mask{coef2=h(10*N+1:11*N)}
      mask prompts: coef2 "Coefficients"
  Cross C12 : Subsystem  mask{coef2=h(11*N+1:12*N)}
      mask prompts: coef2 "Coefficients"
  Cross C13 : Subsystem  mask{coef2=h(12*N+1:13*N)}
      mask prompts: coef2 "Coefficients"
  Cross C14 : Subsystem  mask{coef2=h(13*N+1:14*N)}
      mask prompts: coef2 "Coefficients"
  Cross C15 : Subsystem  mask{coef2=h(14*N+1:15*N)}
      mask prompts: coef2 "Coefficients"
  Cross C16 : Subsystem  mask{coef2=h(15*N+1:16*N)}
      mask prompts: coef2 "Coefficients"
  Cross C2 : Subsystem  mask{coef2=h(1*N+1:2*N)}
      mask prompts: coef2 "Coefficients"
  Cross C3 : Subsystem  mask{coef2=h(2*N+1:3*N)}
      mask prompts: coef2 "Coefficients"
  Cross C4 : Subsystem  mask{coef2=h(3*N+1:4*N)}
      mask prompts: coef2 "Coefficients"
  Cross C5 : Subsystem  mask{coef2=h(4*N+1:5*N)}
      mask prompts: coef2 "Coefficients"
  Cross C6 : Subsystem  mask{coef2=h(5*N+1:6*N)}
      mask prompts: coef2 "Coefficients"
  Cross C7 : Subsystem  mask{coef2=h(6*N+1:7*N)}
      mask prompts: coef2 "Coefficients"
  Cross C8 : Subsystem  mask{coef2=h(7*N+1:8*N)}
      mask prompts: coef2 "Coefficients"
  Cross C9 : Subsystem  mask{coef2=h(8*N+1:9*N)}
      mask prompts: coef2 "Coefficients"
  Delay{1,3..4,6,8,12..13,15} (8 alike) : xbsIndex_r4/Delay  Orientation=down  en=off  latency=N-1  dbl_ovrd=off  reg_retiming=off  block_type=delay
      settings: infoedit=Hardware notes: A delay line is a chain, each link of which is an SRL16 followed by a flip-flop. If register retiming is enabled, the delay line is a chain of flip-flops.  xl_area=[2 4 0 4 0 0 0]  block_version=VER_STRING_GOES_HERE
  Delay10 : xbsIndex_r4/Delay  Orientation=down  en=off  latency=((N-1)*12)-1  dbl_ovrd=off  reg_retiming=off  block_type=delay
      settings: infoedit=Hardware notes: A delay line is a chain, each link of which is an SRL16 followed by a flip-flop. If register retiming is enabled, the delay line is a chain of flip-flops.  xl_area=[9 15 0 15 0 0 0]  block_version=VER_STRING_GOES_HERE
  Delay11 : xbsIndex_r4/Delay  Orientation=down  en=off  latency=((N-1)*14)-1  dbl_ovrd=off  reg_retiming=off  block_type=delay
      settings: infoedit=Hardware notes: A delay line is a chain, each link of which is an SRL16 followed by a flip-flop. If register retiming is enabled, the delay line is a chain of flip-flops.  xl_area=[9 15 0 15 0 0 0]  block_version=VER_STRING_GOES_HERE
  Delay14 : xbsIndex_r4/Delay  Orientation=down  en=off  latency=((N-1)*2)-1  dbl_ovrd=off  reg_retiming=off  block_type=delay
      settings: infoedit=Hardware notes: A delay line is a chain, each link of which is an SRL16 followed by a flip-flop. If register retiming is enabled, the delay line is a chain of flip-flops.  xl_area=[3 5 0 5 0 0 0]  block_version=VER_STRING_GOES_HERE
  Delay2 : xbsIndex_r4/Delay  Orientation=down  en=off  latency=((N-1)*4)-1  dbl_ovrd=off  reg_retiming=off  block_type=delay
      settings: infoedit=Hardware notes: A delay line is a chain, each link of which is an SRL16 followed by a flip-flop. If register retiming is enabled, the delay line is a chain of flip-flops.  xl_area=[3 5 0 5 0 0 0]  block_version=VER_STRING_GOES_HERE
  Delay5 : xbsIndex_r4/Delay  Orientation=down  en=off  latency=((N-1)*6)-1  dbl_ovrd=off  reg_retiming=off  block_type=delay
      settings: infoedit=Hardware notes: A delay line is a chain, each link of which is an SRL16 followed by a flip-flop. If register retiming is enabled, the delay line is a chain of flip-flops.  xl_area=[6 10 0 10 0 0 0]  block_version=VER_STRING_GOES_HERE
  Delay7 : xbsIndex_r4/Delay  Orientation=down  en=off  latency=((N-1)*8)-1  dbl_ovrd=off  reg_retiming=off  block_type=delay
      settings: infoedit=Hardware notes: A delay line is a chain, each link of which is an SRL16 followed by a flip-flop. If register retiming is enabled, the delay line is a chain of flip-flops.  xl_area=[6 10 0 10 0 0 0]  block_version=VER_STRING_GOES_HERE
  Delay9 : xbsIndex_r4/Delay  Orientation=down  en=off  latency=((N-1)*10)-1  dbl_ovrd=off  reg_retiming=off  block_type=delay
      settings: infoedit=Hardware notes: A delay line is a chain, each link of which is an SRL16 followed by a flip-flop. If register retiming is enabled, the delay line is a chain of flip-flops.  xl_area=[9 15 0 15 0 0 0]  block_version=VER_STRING_GOES_HERE
  FSM : Subsystem
  Gateway Out6 : xbsIndex_r4/Gateway Out  hdl_port=off  timing_constraint=None  locs_specified=off  LOCs={}  block_type=gatewayout
      settings: infoedit="Gateway out block.  Converts Xilinx fixed point inputs into ouputs of type Simulink integer, double, or fixed point.<P><P>Hardware notes:  In hardware these blocks become top level output ports or are discarded, depending on how they are configured."  xl_area=[0 0 0 0 0 0 0]  block_version=VER_STRING_GOES_HERE
  Gateway Out7 : xbsIndex_r4/Gateway Out  hdl_port=off  timing_constraint=None  locs_specified=off  LOCs={}  block_type=gatewayout
      settings: infoedit="Gateway out block.  Converts Xilinx fixed point inputs into ouputs of type Simulink integer, double, or fixed point.<P><P>Hardware notes:  In hardware these blocks become top level output ports or are discarded, depending on how they are configured."  xl_area=[0 0 0 0 0 0 0]  block_version=VER_STRING_GOES_HERE
  Gateway Out8 : xbsIndex_r4/Gateway Out  hdl_port=off  timing_constraint=None  locs_specified=off  LOCs={}  block_type=gatewayout
      settings: infoedit="Gateway out block.  Converts Xilinx fixed point inputs into ouputs of type Simulink integer, double, or fixed point.<P><P>Hardware notes:  In hardware these blocks become top level output ports or are discarded, depending on how they are configured."  xl_area=[0 0 0 0 0 0 0]  block_version=VER_STRING_GOES_HERE
  Long Corr Scope2 : Scope  NumInputPorts=3
      settings: YMin=-0.35~-0.175~0  YMax=0.35~0.225~1300  SaveName=ScopeData8
  Up Sample : xbsIndex_r4/Up Sample  sample_ratio=4  copy_samples=on  dbl_ovrd=off  block_type=usamp
      settings: infoedit="Up samples input data.  Inserted values  can be zeros or copies of the most recent input sample.<P><P>Hardware notes: No hardware is needed if inserted values are copies of the input sample; otherwise, a mux and single bit flip-flop are used."  block_version=8.2.02
  y : Outport
  wires:
  Up Sample/1 -> Cross C1/1(xn)
  Gateway Out8/1 -> Long Corr Scope2/3
  Gateway Out7/1 -> Long Corr Scope2/2
  Gateway Out6/1 -> Long Corr Scope2/1
  AddSub14/1 -> Gateway Out6/1, AddSub9/2
  Delay11/1 -> AddSub14/2
  Delay10/1 -> AddSub14/1
  AddSub11/1 -> Delay11/1
  AddSub10/1 -> Delay10/1
  Delay15/1 -> AddSub11/2
  Delay12/1 -> AddSub10/2
  Cross C16/1(yn) -> Delay15/1
  Cross C14/1(yn) -> Delay12/1
  Cross C15/1(yn) -> AddSub11/1
  Cross C15/2(xnz) -> Cross C16/1(xn)
  Cross C14/2(xnz) -> Cross C15/1(xn)
  Cross C13/2(xnz) -> Cross C14/1(xn)
  x/1 -> Up Sample/1
  Cross C2/1(yn) -> Delay3/1
  Cross C1/2(xnz) -> Cross C2/1(xn)
  FSM/1(Data Addr) -> Cross C2/2(DAddr), Cross C3/2(DAddr), Cross C4/2(DAddr), Cross C5/2(DAddr), Cross C6/2(DAddr), Cross C8/2(DAddr), Cross C7/2(DAddr), Cross C1/2(DAddr), Cross C9/2(DAddr), Cross C10/2(DAddr), Cross C11/2(DAddr), Cross C12/2(DAddr), Cross C13/2(DAddr), Cross C14/2(DAddr), Cross C16/2(DAddr), Cross C15/2(DAddr)
  FSM/2(Coef Addr) -> Cross C2/3(CAddr), Cross C3/3(CAddr), Cross C4/3(CAddr), Cross C5/3(CAddr), Cross C6/3(CAddr), Cross C8/3(CAddr), Cross C7/3(CAddr), Cross C1/3(CAddr), Cross C9/3(CAddr), Cross C10/3(CAddr), Cross C11/3(CAddr), Cross C12/3(CAddr), Cross C13/3(CAddr), Cross C14/3(CAddr), Cross C16/3(CAddr), Cross C15/3(CAddr)
  FSM/3(load) -> Cross C2/4(LD), Cross C3/4(LD), Cross C4/4(LD), Cross C5/4(LD), Cross C6/4(LD), Cross C8/4(LD), Cross C7/4(LD), Cross C1/4(LD), Cross C9/4(LD), Cross C10/4(LD), Cross C11/4(LD), Cross C12/4(LD), Cross C13/4(LD), Cross C14/4(LD), Cross C16/4(LD), Cross C15/4(LD)
  Cross C2/2(xnz) -> Cross C3/1(xn)
  Cross C3/2(xnz) -> Cross C4/1(xn)
  Cross C3/1(yn) -> AddSub12/1
  Cross C4/2(xnz) -> Cross C5/1(xn)
  Cross C4/1(yn) -> Delay13/1
  Cross C5/2(xnz) -> Cross C6/1(xn)
  Cross C6/2(xnz) -> Cross C7/1(xn)
  [xnz] -> Cross C9/1(xn)
  Cross C9/2(xnz) -> Cross C10/1(xn)
  Cross C10/2(xnz) -> Cross C11/1(xn)
  Cross C11/2(xnz) -> Cross C12/1(xn)
  Cross C12/2(xnz) -> Cross C13/1(xn)
  Delay13/1 -> AddSub12/2
  AddSub12/1 -> Delay14/1
  Delay3/1 -> AddSub/2
  Cross C1/1(yn) -> AddSub/1
  Cross C5/1(yn) -> AddSub1/1
  Delay1/1 -> AddSub1/2
  AddSub1/1 -> Delay2/1
  Cross C6/1(yn) -> Delay1/1
  Cross C7/2(xnz) -> Cross C8/1(xn)
  Delay4/1 -> AddSub3/2
  AddSub3/1 -> Delay5/1
  Cross C7/1(yn) -> AddSub3/1
  Cross C8/1(yn) -> Delay4/1
  Cross C9/1(yn) -> AddSub5/1
  Delay6/1 -> AddSub5/2
  AddSub5/1 -> Delay7/1
  Cross C10/1(yn) -> Delay6/1
  Cross C8/2(xnz) -> [xnz]
  Delay14/1 -> AddSub13/2
  AddSub/1 -> AddSub13/1
  Delay2/1 -> AddSub2/1
  Delay5/1 -> AddSub2/2
  AddSub13/1 -> AddSub4/1
  AddSub2/1 -> AddSub4/2
  AddSub4/1 -> AddSub8/1
  Cross C11/1(yn) -> AddSub7/1
  Delay8/1 -> AddSub7/2
  AddSub7/1 -> Delay9/1
  Cross C12/1(yn) -> Delay8/1
  Delay7/1 -> AddSub6/1
  Delay9/1 -> AddSub6/2
  AddSub8/1 -> y/1
  Cross C13/1(yn) -> AddSub10/1
  AddSub6/1 -> Gateway Out7/1, AddSub9/1
  AddSub9/1 -> AddSub8/2, Gateway Out8/1
